simulink model "TosAeb"  (R2021a)
settings: Solver=FixedStepDiscrete  StartTime=0.0  StopTime=inf  FixedStep=0.01  RelTol=1e-3
undefined symbols (not defined in the file or in its folder): ACCModSts, AccSts1, AEB_ObjectMovingOrientation, AEB_ObjectMovingState, CN_BTCActive, CN_CurrentGearR, CN_CurrentGearR_DHT, CN_PTCActive, CN_VDCActive, CN_VSC_EspOff, CN_VSC_FCW_AEB_Error, CN_VSC_FCW_AEB_NoDispay, CN_VSC_FCW_AEB_PerfmDgdtn, CN_VSC_FCW_AEB_TempUnAvlbl, CN_VSC_VehStandstill, CNaccelAvoidMax, CNaccelAvoidMin, CNbrakePedalPress, CNcomfortStrLeftAccelMax, CNcomfortStrLeftAccelMin, CNcomfortStrRightAccelMax, CNcomfortStrRightAccelMin, CNDrvDoorOpen, CNDrvModeEco, CNDrvModeNorm, CNDrvModeOther, CNDrvModeSport, CNDrvSBRreminder, CNNull, CNobjInvalidID, CNPassDoorOpen, CNPassSBRreminder, CNPEBSAccelNegInf, CNPEBSAccelNull, CNPEBSTimeInf, CNPEBSTimeNull, CNSTOPMAX, CNSTOPNULL, CNsubjWidth, CNSwtOFF, CNSwtON, CNTTCMAX, CNTTCNULL, CNVehConfigAEBCycNone, CNVehConfigAEBNone, CNVehConfigAEBPedNone, CNVehConfigFCWNone, CNVehConfigJANone, CollisionSeverity, FALSE, FCWSnvty, JACycObjCalcInfoDefaultParams, JAPedObjCalcInfoDefaultParams, JAVehObjCalcInfoDefaultParams, latCycObjCalcInfoDefaultParams, latPedObjCalcInfoDefaultParams, longObjCalcInfoDefaultParams, ModSts, movingStatus, ObjectType ... (+1112)
libraries: simulink x3031, AEBSFunctionLib x50, Ta_Library x33, AEBSStateMachineLib x23, Mylibrary x12, simulink_extras x7, ObjSelect_lib x3, dspstat3 x2
(part 83 of 102)

== system TosAeb/FcwAebJa_Step_sys/ALG_AEBSystem/ALG_AEBController/AEB_FuncImpManagementApp/FuncManeuArbCalc/brkFuncManeuArbCalc/axTgtReqValueCalc1/axTgtReqValueCalc4AebControl/aDesiredCalc/If Action Subsystem1/accelAvoidLowLvlBrkCalc/Situation1/Case2 ==
  note: vEgo^2
      | aAvoid = ----------------------------------
      |                   vObj^2
      |                  ---------  - 2(dRel - dScure)
      |                   aObj
  subjVel : Inport
  vxAbsObj : Inport  Port=2
  axAbsObj : Inport  Port=3
  dxEgoBrake : Inport  Port=4
  Action Port : ActionPort  ActionPortLabel=else
  Divide : Product  Inputs=*/
  Divide1 : Product  Inputs=*/
  Gain : Gain  Gain=2
  Math Function : Math  Operator=square  SignedPower=on  SaturateOnIntegerOverflow=on
  Math Function1 : Math  Operator=square  SignedPower=on  SaturateOnIntegerOverflow=on
  Subtract : Sum  Inputs=+-
  accelAvoid : Outport
  wires:
  Divide1/1 -> accelAvoid/1
  Math Function1/1 -> Divide1/1
  Subtract/1 -> Divide1/2
  subjVel/1 -> Math Function1/1
  Gain/1 -> Subtract/2
  dxEgoBrake/1 -> Gain/1
  Divide/1 -> Subtract/1
  axAbsObj/1 -> Divide/2
  Math Function/1 -> Divide/1
  vxAbsObj/1 -> Math Function/1

== system TosAeb/FcwAebJa_Step_sys/ALG_AEBSystem/ALG_AEBController/AEB_FuncImpManagementApp/FuncManeuArbCalc/brkFuncManeuArbCalc/axTgtReqValueCalc1/axTgtReqValueCalc4AebControl/aDesiredCalc/If Action Subsystem1/accelAvoidLowLvlBrkCalc/Situation1/collisionFirstFlgCalc ==
  note: Calculate Ego and Obj Collision Time and
      |  Determine if the Collision Time is less than the object stop time
  dxEgoBrake : Inport
  vx : Inport  Port=2
  tObjStop : Inport  Port=3
  CNTTCMAX : Constant  Value=CNTTCMAX
  Divide : Product  Inputs=*/
  Gain : Gain  Gain=-1
  Gain1 : Gain  Gain=2
  MinMax : MinMax  Inputs=2
  Relational Operator : RelationalOperator  Operator=<  OutDataTypeStr=boolean  RndMeth=Simplest
  collisionFirstFlg : Outport
  wires:
  CNTTCMAX/1 -> MinMax/2
  MinMax/1 -> Relational Operator/1
  Relational Operator/1 -> collisionFirstFlg/1  "collisionFirstFlg"
  tObjStop/1 -> Relational Operator/2
  Gain1/1 -> MinMax/1
  Gain/1 -> Divide/2
  vx/1 -> Gain/1
  dxEgoBrake/1 -> Divide/1
  Divide/1 -> Gain1/1

== system TosAeb/FcwAebJa_Step_sys/ALG_AEBSystem/ALG_AEBController/AEB_JaObjCalcApp/JAPedTgtInfoCalc/JAPedObjCalcInfoCalc/JAPedObjInfoCalc/ObjCrossFlgCal/CollisionProbCalc/Subsystem/PreCrossFlgCalcFlow/VectorCrossAlgorithm/If Action Subsystem1/Subsystem ==
  temp_u : Inport  OutDataTypeStr=single
  temp_t : Inport  Port=2  OutDataTypeStr=single
  vectorMulit : Inport  Port=3
  Compare To Constant : simulink/Logic and Bit Operations/Compare To Constant  relop=>=  const=0  OutDataTypeStr=boolean
  Compare To Constant1 : simulink/Logic and Bit Operations/Compare To Constant  relop=<=  const=1  OutDataTypeStr=boolean
  Compare To Constant2 : simulink/Logic and Bit Operations/Compare To Constant  relop=>=  const=0  OutDataTypeStr=boolean
  Compare To Constant3 : simulink/Logic and Bit Operations/Compare To Constant  relop=<=  const=1  OutDataTypeStr=boolean
  Divide : Product  Inputs=*/
  Divide1 : Product  Inputs=*/
  Logical Operator : Logic  Inputs=4  AllPortsSameDT=off  OutDataTypeStr=boolean
  CrossFlg : Outport
  wires:
  vectorMulit/1 -> Divide1/2, Divide/2
  temp_t/1 -> Divide/1
  temp_u/1 -> Divide1/1
  Divide1/1 -> Compare To Constant1/1, Compare To Constant/1
  Divide/1 -> Compare To Constant3/1, Compare To Constant2/1
  Compare To Constant1/1 -> Logical Operator/2
  Compare To Constant2/1 -> Logical Operator/3
  Compare To Constant/1 -> Logical Operator/1
  Compare To Constant3/1 -> Logical Operator/4
  Logical Operator/1 -> CrossFlg/1

== system TosAeb/FcwAebJa_Step_sys/ALG_AEBSystem/ALG_AEBController/AEB_JaObjCalcApp/JAPedTgtInfoCalc/JAPedObjCalcInfoCalc/JAPedObjInfoCalc/ObjCrossFlgCal/CollisionProbCalc/Subsystem/PreCrossFlgCalcFlow/VectorCrossAlgorithm/If Action Subsystem1/t ==
  m2 : Inport  OutDataTypeStr=single
  m1 : Inport  Port=2  OutDataTypeStr=single
  y1 : Inport  Port=3  OutDataTypeStr=single
  n1 : Inport  Port=4  OutDataTypeStr=single
  n2 : Inport  Port=5  OutDataTypeStr=single
  x1 : Inport  Port=6  OutDataTypeStr=single
  Product : Product  OutDataTypeStr=single
  Product1 : Product  OutDataTypeStr=single
  Subtract{1..5} (5 alike) : Sum  Inputs=+-  OutDataTypeStr=single
  temp_t : Outport
  wires:
  m2/1 -> Subtract2/1
  m1/1 -> Subtract2/2, Subtract1/2
  y1/1 -> Subtract3/1
  Subtract2/1 -> Product/1
  Subtract3/1 -> Product/2
  n2/1 -> Subtract4/1
  n1/1 -> Subtract4/2, Subtract3/2
  x1/1 -> Subtract1/1
  Subtract4/1 -> Product1/1
  Subtract1/1 -> Product1/2
  Product/1 -> Subtract5/1
  Product1/1 -> Subtract5/2
  Subtract5/1 -> temp_t/1

== system TosAeb/FcwAebJa_Step_sys/ALG_AEBSystem/ALG_AEBController/AEB_JaObjCalcApp/JAPedTgtInfoCalc/JAPedObjCalcInfoCalc/JAPedObjInfoCalc/ObjCrossFlgCal/CollisionProbCalc/Subsystem/PreCrossFlgCalcFlow/VectorCrossAlgorithm/If Action Subsystem1/u ==
  x2 : Inport  OutDataTypeStr=single
  x1 : Inport  Port=2  OutDataTypeStr=single
  y1 : Inport  Port=3  OutDataTypeStr=single
  n1 : Inport  Port=4  OutDataTypeStr=single
  y2 : Inport  Port=5  OutDataTypeStr=single
  m1 : Inport  Port=6  OutDataTypeStr=single
  Product : Product  OutDataTypeStr=single
  Product1 : Product  OutDataTypeStr=single
  Subtract{1..5} (5 alike) : Sum  Inputs=+-  OutDataTypeStr=single
  temp_u : Outport
  wires:
  Product1/1 -> Subtract5/2
  Product/1 -> Subtract5/1
  Subtract1/1 -> Product1/2
  Subtract4/1 -> Product1/1
  n1/1 -> Subtract3/2
  y2/1 -> Subtract4/1
  Subtract3/1 -> Product/2
  Subtract2/1 -> Product/1
  y1/1 -> Subtract4/2, Subtract3/1
  x1/1 -> Subtract1/1, Subtract2/2
  x2/1 -> Subtract2/1
  m1/1 -> Subtract1/2
  Subtract5/1 -> temp_u/1

== system TosAeb/FcwAebJa_Step_sys/ALG_AEBSystem/ALG_AEBController/AEB_JaObjCalcApp/JAVehTgtInfoCalc/AEBJAVehObjCalc/JAVehObjInfoCalc/ObjCrossFlgCal/CollisionProbCalc/Subsystem/PreCrossFlgCalcFlow/VectorCrossAlgorithm/If Action Subsystem1/Subsystem ==
  temp_u : Inport  OutDataTypeStr=single
  temp_t : Inport  Port=2  OutDataTypeStr=single
  vectorMulit : Inport  Port=3
  Compare To Constant : simulink/Logic and Bit Operations/Compare To Constant  relop=>=  const=0  OutDataTypeStr=boolean
  Compare To Constant1 : simulink/Logic and Bit Operations/Compare To Constant  relop=<=  const=1  OutDataTypeStr=boolean
  Compare To Constant2 : simulink/Logic and Bit Operations/Compare To Constant  relop=>=  const=0  OutDataTypeStr=boolean
  Compare To Constant3 : simulink/Logic and Bit Operations/Compare To Constant  relop=<=  const=1  OutDataTypeStr=boolean
  Divide : Product  Inputs=*/
  Divide1 : Product  Inputs=*/
  Logical Operator : Logic  Inputs=4  AllPortsSameDT=off  OutDataTypeStr=boolean
  CrossFlg : Outport
  wires:
  vectorMulit/1 -> Divide1/2, Divide/2
  temp_t/1 -> Divide/1
  temp_u/1 -> Divide1/1
  Divide1/1 -> Compare To Constant1/1, Compare To Constant/1
  Divide/1 -> Compare To Constant3/1, Compare To Constant2/1
  Compare To Constant1/1 -> Logical Operator/2
  Compare To Constant2/1 -> Logical Operator/3
  Compare To Constant/1 -> Logical Operator/1
  Compare To Constant3/1 -> Logical Operator/4
  Logical Operator/1 -> CrossFlg/1

== system TosAeb/FcwAebJa_Step_sys/ALG_AEBSystem/ALG_AEBController/AEB_JaObjCalcApp/JAVehTgtInfoCalc/AEBJAVehObjCalc/JAVehObjInfoCalc/ObjCrossFlgCal/CollisionProbCalc/Subsystem/PreCrossFlgCalcFlow/VectorCrossAlgorithm/If Action Subsystem1/t ==
  m2 : Inport  OutDataTypeStr=single
  m1 : Inport  Port=2  OutDataTypeStr=single
  y1 : Inport  Port=3  OutDataTypeStr=single
  n1 : Inport  Port=4  OutDataTypeStr=single
  n2 : Inport  Port=5  OutDataTypeStr=single
  x1 : Inport  Port=6  OutDataTypeStr=single
  Product : Product  OutDataTypeStr=single
  Product1 : Product  OutDataTypeStr=single
  Subtract{1..5} (5 alike) : Sum  Inputs=+-  OutDataTypeStr=single
  temp_t : Outport
  wires:
  m2/1 -> Subtract2/1
  m1/1 -> Subtract2/2, Subtract1/2
  y1/1 -> Subtract3/1
  Subtract2/1 -> Product/1
  Subtract3/1 -> Product/2
  n2/1 -> Subtract4/1
  n1/1 -> Subtract4/2, Subtract3/2
  x1/1 -> Subtract1/1
  Subtract4/1 -> Product1/1
  Subtract1/1 -> Product1/2
  Product/1 -> Subtract5/1
  Product1/1 -> Subtract5/2
  Subtract5/1 -> temp_t/1

== system TosAeb/FcwAebJa_Step_sys/ALG_AEBSystem/ALG_AEBController/AEB_JaObjCalcApp/JAVehTgtInfoCalc/AEBJAVehObjCalc/JAVehObjInfoCalc/ObjCrossFlgCal/CollisionProbCalc/Subsystem/PreCrossFlgCalcFlow/VectorCrossAlgorithm/If Action Subsystem1/u ==
  x2 : Inport  OutDataTypeStr=single
  x1 : Inport  Port=2  OutDataTypeStr=single
  y1 : Inport  Port=3  OutDataTypeStr=single
  n1 : Inport  Port=4  OutDataTypeStr=single
  y2 : Inport  Port=5  OutDataTypeStr=single
  m1 : Inport  Port=6  OutDataTypeStr=single
  Product : Product  OutDataTypeStr=single
  Product1 : Product  OutDataTypeStr=single
  Subtract{1..5} (5 alike) : Sum  Inputs=+-  OutDataTypeStr=single
  temp_u : Outport
  wires:
  Product1/1 -> Subtract5/2
  Product/1 -> Subtract5/1
  Subtract1/1 -> Product1/2
  Subtract4/1 -> Product1/1
  n1/1 -> Subtract3/2
  y2/1 -> Subtract4/1
  Subtract3/1 -> Product/2
  Subtract2/1 -> Product/1
  y1/1 -> Subtract4/2, Subtract3/1
  x1/1 -> Subtract1/1, Subtract2/2
  x2/1 -> Subtract2/1
  m1/1 -> Subtract1/2
  Subtract5/1 -> temp_u/1

== system TosAeb/FcwAebJa_Step_sys/ALG_AEBSystem/ALG_AEBController/AEB_JaObjCalcApp/JaCycTgtInfoCalc/JaCycObjCalcInfoCalc/JaCycObjInfoCalc/ObjCrossFlgCal/CollisionProbCalc/Subsystem/PreCrossFlgCalcFlow/VectorCrossAlgorithm/If Action Subsystem1/Subsystem ==
  temp_u : Inport  OutDataTypeStr=single
  temp_t : Inport  Port=2  OutDataTypeStr=single
  vectorMulit : Inport  Port=3
  Compare To Constant : simulink/Logic and Bit Operations/Compare To Constant  relop=>=  const=0  OutDataTypeStr=boolean
  Compare To Constant1 : simulink/Logic and Bit Operations/Compare To Constant  relop=<=  const=1  OutDataTypeStr=boolean
  Compare To Constant2 : simulink/Logic and Bit Operations/Compare To Constant  relop=>=  const=0  OutDataTypeStr=boolean
  Compare To Constant3 : simulink/Logic and Bit Operations/Compare To Constant  relop=<=  const=1  OutDataTypeStr=boolean
  Divide : Product  Inputs=*/
  Divide1 : Product  Inputs=*/
  Logical Operator : Logic  Inputs=4  AllPortsSameDT=off  OutDataTypeStr=boolean
  CrossFlg : Outport
  wires:
  vectorMulit/1 -> Divide1/2, Divide/2
  temp_t/1 -> Divide/1
  temp_u/1 -> Divide1/1
  Divide1/1 -> Compare To Constant1/1, Compare To Constant/1
  Divide/1 -> Compare To Constant3/1, Compare To Constant2/1
  Compare To Constant1/1 -> Logical Operator/2
  Compare To Constant2/1 -> Logical Operator/3
  Compare To Constant/1 -> Logical Operator/1
  Compare To Constant3/1 -> Logical Operator/4
  Logical Operator/1 -> CrossFlg/1

== system TosAeb/FcwAebJa_Step_sys/ALG_AEBSystem/ALG_AEBController/AEB_JaObjCalcApp/JaCycTgtInfoCalc/JaCycObjCalcInfoCalc/JaCycObjInfoCalc/ObjCrossFlgCal/CollisionProbCalc/Subsystem/PreCrossFlgCalcFlow/VectorCrossAlgorithm/If Action Subsystem1/t ==
  m2 : Inport  OutDataTypeStr=single
  m1 : Inport  Port=2  OutDataTypeStr=single
  y1 : Inport  Port=3  OutDataTypeStr=single
  n1 : Inport  Port=4  OutDataTypeStr=single
  n2 : Inport  Port=5  OutDataTypeStr=single
  x1 : Inport  Port=6  OutDataTypeStr=single
  Product : Product  OutDataTypeStr=single
  Product1 : Product  OutDataTypeStr=single
  Subtract{1..5} (5 alike) : Sum  Inputs=+-  OutDataTypeStr=single
  temp_t : Outport
  wires:
  m2/1 -> Subtract2/1
  m1/1 -> Subtract2/2, Subtract1/2
  y1/1 -> Subtract3/1
  Subtract2/1 -> Product/1
  Subtract3/1 -> Product/2
  n2/1 -> Subtract4/1
  n1/1 -> Subtract4/2, Subtract3/2
  x1/1 -> Subtract1/1
  Subtract4/1 -> Product1/1
  Subtract1/1 -> Product1/2
  Product/1 -> Subtract5/1
  Product1/1 -> Subtract5/2
  Subtract5/1 -> temp_t/1

== system TosAeb/FcwAebJa_Step_sys/ALG_AEBSystem/ALG_AEBController/AEB_JaObjCalcApp/JaCycTgtInfoCalc/JaCycObjCalcInfoCalc/JaCycObjInfoCalc/ObjCrossFlgCal/CollisionProbCalc/Subsystem/PreCrossFlgCalcFlow/VectorCrossAlgorithm/If Action Subsystem1/u ==
  x2 : Inport  OutDataTypeStr=single
  x1 : Inport  Port=2  OutDataTypeStr=single
  y1 : Inport  Port=3  OutDataTypeStr=single
  n1 : Inport  Port=4  OutDataTypeStr=single
  y2 : Inport  Port=5  OutDataTypeStr=single
  m1 : Inport  Port=6  OutDataTypeStr=single
  Product : Product  OutDataTypeStr=single
  Product1 : Product  OutDataTypeStr=single
  Subtract{1..5} (5 alike) : Sum  Inputs=+-  OutDataTypeStr=single
  temp_u : Outport
  wires:
  Product1/1 -> Subtract5/2
  Product/1 -> Subtract5/1
  Subtract1/1 -> Product1/2
  Subtract4/1 -> Product1/1
  n1/1 -> Subtract3/2
  y2/1 -> Subtract4/1
  Subtract3/1 -> Product/2
  Subtract2/1 -> Product/1
  y1/1 -> Subtract4/2, Subtract3/1
  x1/1 -> Subtract1/1, Subtract2/2
  x2/1 -> Subtract2/1
  m1/1 -> Subtract1/2
  Subtract5/1 -> temp_u/1

== system TosAeb/FcwAebJa_Step_sys/ALG_AEBSystem/ALG_AEBController/AEB_LatObjInfoCalcApp/LatCycObjCalc/LatCycObjCalc/latCycObjInfoCalc/ObjColiisionRiskProbCalc/CollisionRiskProbCalc/ObjReginCorrCalc/AccelXYMaxMinCorrCalc/AccelXMaxMinCorrCalc/ObjDwn2UpMoving ==
  AccelMaxX : Inport
  AccelMinX : Inport  Port=2
  Action Port : ActionPort  ActionPortLabel=elseif(u1 > 0)
  AccelXMaxCorr : Outport
  AccelXMinCorr : Outport  Port=2
  wires:
  AccelMaxX/1 -> AccelXMaxCorr/1
  AccelMinX/1 -> AccelXMinCorr/1

== system TosAeb/FcwAebJa_Step_sys/ALG_AEBSystem/ALG_AEBController/AEB_LatObjInfoCalcApp/LatCycObjCalc/LatCycObjCalc/latCycObjInfoCalc/ObjColiisionRiskProbCalc/CollisionRiskProbCalc/ObjReginCorrCalc/AccelXYMaxMinCorrCalc/AccelXMaxMinCorrCalc/ObjStanding ==
  Action Port : ActionPort  ActionPortLabel=else
  Constant : Constant  Value=CNNull
  Constant1 : Constant  Value=CNNull
  AccelXMaxCorr : Outport
  AccelXMinCorr : Outport  Port=2
  wires:
  Constant1/1 -> AccelXMinCorr/1
  Constant/1 -> AccelXMaxCorr/1

== system TosAeb/FcwAebJa_Step_sys/ALG_AEBSystem/ALG_AEBController/AEB_LatObjInfoCalcApp/LatCycObjCalc/LatCycObjCalc/latCycObjInfoCalc/ObjColiisionRiskProbCalc/CollisionRiskProbCalc/ObjReginCorrCalc/AccelXYMaxMinCorrCalc/AccelXMaxMinCorrCalc/ObjUp2DwnMoving ==
  AccelMaxX : Inport
  AccelMinX : Inport  Port=2
  Action Port : ActionPort  ActionPortLabel=if(u1 < 0)
  AccelXMaxCorr : Outport
  AccelXMinCorr : Outport  Port=2
  wires:
  AccelMaxX/1 -> AccelXMaxCorr/1
  AccelMinX/1 -> AccelXMinCorr/1

== system TosAeb/FcwAebJa_Step_sys/ALG_AEBSystem/ALG_AEBController/AEB_LatObjInfoCalcApp/LatCycObjCalc/LatCycObjCalc/latCycObjInfoCalc/ObjColiisionRiskProbCalc/CollisionRiskProbCalc/ObjReginCorrCalc/AccelXYMaxMinCorrCalc/AccelYMaxMinCorrCalc/ObjLeft2RightMoving ==
  AccelYMax : Inport
  AccelYMin : Inport  Port=2
  Action Port : ActionPort  ActionPortLabel=if(u1 < 0)
  AccelYMaxCorr : Outport
  AccelYMinCorr : Outport  Port=2
  wires:
  AccelYMax/1 -> AccelYMinCorr/1
  AccelYMin/1 -> AccelYMaxCorr/1

== system TosAeb/FcwAebJa_Step_sys/ALG_AEBSystem/ALG_AEBController/AEB_LatObjInfoCalcApp/LatCycObjCalc/LatCycObjCalc/latCycObjInfoCalc/ObjColiisionRiskProbCalc/CollisionRiskProbCalc/ObjReginCorrCalc/AccelXYMaxMinCorrCalc/AccelYMaxMinCorrCalc/ObjRight2LeftMoving ==
  AccelYMax : Inport
  AccelYMin : Inport  Port=2
  Action Port : ActionPort  ActionPortLabel=elseif(u1 > 0)
  AccelYMaxCorr : Outport
  AccelYMinCorr : Outport  Port=2
  wires:
  AccelYMin/1 -> AccelYMinCorr/1
  AccelYMax/1 -> AccelYMaxCorr/1

== system TosAeb/FcwAebJa_Step_sys/ALG_AEBSystem/ALG_AEBController/AEB_LatObjInfoCalcApp/LatCycObjCalc/LatCycObjCalc/latCycObjInfoCalc/ObjColiisionRiskProbCalc/CollisionRiskProbCalc/ObjReginCorrCalc/AccelXYMaxMinCorrCalc/AccelYMaxMinCorrCalc/ObjStanding ==
  Action Port : ActionPort  ActionPortLabel=else
  Constant : Constant  Value=CNNull
  Constant1 : Constant  Value=CNNull
  AccelYMaxCorr : Outport
  AccelYMinCorr : Outport  Port=2
  wires:
  Constant/1 -> AccelYMaxCorr/1
  Constant1/1 -> AccelYMinCorr/1

== system TosAeb/FcwAebJa_Step_sys/ALG_AEBSystem/ALG_AEBController/AEB_LatObjInfoCalcApp/LatPedObjCalc/LatPedObjCalc/latPedObjInfoCalc/ObjColiisionRiskProbCalc/CollisionRiskProbCalc/ObjReginCorrCalc/AccelXYMaxMinCorrCalc/AccelXMaxMinCorrCalc/ObjDwn2UpMoving ==
  AccelMaxX : Inport  OutDataTypeStr=single
  AccelMinX : Inport  Port=2  OutDataTypeStr=single
  Action Port : ActionPort  ActionPortLabel=elseif(u1 > 0)
  AccelXMaxCorr : Outport
  AccelXMinCorr : Outport  Port=2
  wires:
  AccelMinX/1 -> AccelXMinCorr/1
  AccelMaxX/1 -> AccelXMaxCorr/1

== system TosAeb/FcwAebJa_Step_sys/ALG_AEBSystem/ALG_AEBController/AEB_LatObjInfoCalcApp/LatPedObjCalc/LatPedObjCalc/latPedObjInfoCalc/ObjColiisionRiskProbCalc/CollisionRiskProbCalc/ObjReginCorrCalc/AccelXYMaxMinCorrCalc/AccelXMaxMinCorrCalc/ObjStanding ==
  Action Port : ActionPort  ActionPortLabel=else
  Constant : Constant  Value=0  OutDataTypeStr=single
  Constant1 : Constant  Value=0  OutDataTypeStr=single
  AccelXMaxCorr : Outport
  AccelXMinCorr : Outport  Port=2
  wires:
  Constant/1 -> AccelXMaxCorr/1
  Constant1/1 -> AccelXMinCorr/1

== system TosAeb/FcwAebJa_Step_sys/ALG_AEBSystem/ALG_AEBController/AEB_LatObjInfoCalcApp/LatPedObjCalc/LatPedObjCalc/latPedObjInfoCalc/ObjColiisionRiskProbCalc/CollisionRiskProbCalc/ObjReginCorrCalc/AccelXYMaxMinCorrCalc/AccelXMaxMinCorrCalc/ObjUp2DwnMoving ==
  AccelMaxX : Inport  OutDataTypeStr=single
  AccelMinX : Inport  Port=2  OutDataTypeStr=single
  Action Port : ActionPort  ActionPortLabel=if(u1 < 0)
  AccelXMaxCorr : Outport
  AccelXMinCorr : Outport  Port=2
  wires:
  AccelMaxX/1 -> AccelXMinCorr/1
  AccelMinX/1 -> AccelXMaxCorr/1

== system TosAeb/FcwAebJa_Step_sys/ALG_AEBSystem/ALG_AEBController/AEB_LatObjInfoCalcApp/LatPedObjCalc/LatPedObjCalc/latPedObjInfoCalc/ObjColiisionRiskProbCalc/CollisionRiskProbCalc/ObjReginCorrCalc/AccelXYMaxMinCorrCalc/AccelYMaxMinCorrCalc/ObjLeft2RightMoving ==
  AccelYMax : Inport  OutDataTypeStr=single
  AccelYMin : Inport  Port=2  OutDataTypeStr=single
  Action Port : ActionPort  ActionPortLabel=if(u1 < 0)
  AccelYMaxCorr : Outport
  AccelYMinCorr : Outport  Port=2
  wires:
  AccelYMin/1 -> AccelYMaxCorr/1
  AccelYMax/1 -> AccelYMinCorr/1

== system TosAeb/FcwAebJa_Step_sys/ALG_AEBSystem/ALG_AEBController/AEB_LatObjInfoCalcApp/LatPedObjCalc/LatPedObjCalc/latPedObjInfoCalc/ObjColiisionRiskProbCalc/CollisionRiskProbCalc/ObjReginCorrCalc/AccelXYMaxMinCorrCalc/AccelYMaxMinCorrCalc/ObjRight2LeftMoving ==
  AccelYMax : Inport  OutDataTypeStr=single
  AccelYMin : Inport  Port=2  OutDataTypeStr=single
  Action Port : ActionPort  ActionPortLabel=elseif(u1 > 0)
  AccelYMaxCorr : Outport
  AccelYMinCorr : Outport  Port=2
  wires:
  AccelYMax/1 -> AccelYMaxCorr/1
  AccelYMin/1 -> AccelYMinCorr/1

== system TosAeb/FcwAebJa_Step_sys/ALG_AEBSystem/ALG_AEBController/AEB_LatObjInfoCalcApp/LatPedObjCalc/LatPedObjCalc/latPedObjInfoCalc/ObjColiisionRiskProbCalc/CollisionRiskProbCalc/ObjReginCorrCalc/AccelXYMaxMinCorrCalc/AccelYMaxMinCorrCalc/ObjStanding ==
  Action Port : ActionPort  ActionPortLabel=else
  Constant : Constant  Value=0  OutDataTypeStr=single
  Constant1 : Constant  Value=0  OutDataTypeStr=single
  AccelYMaxCorr : Outport
  AccelYMinCorr : Outport  Port=2
  wires:
  Constant1/1 -> AccelYMinCorr/1
  Constant/1 -> AccelYMaxCorr/1

== system TosAeb/FcwAebJa_Step_sys/ALG_AEBSystem/ALG_AEBController/AEB_LatObjInfoCalcApp/LatPedObjCalc/Subsystem/latPedObjInfoCalc/ObjColiisionRiskProbCalc/CollisionRiskProbCalc/ObjReginCorrCalc/AccelXYMaxMinCorrCalc/AccelXMaxMinCorrCalc/ObjDwn2UpMoving ==
  AccelMaxX : Inport  OutDataTypeStr=single
  AccelMinX : Inport  Port=2  OutDataTypeStr=single
  Action Port : ActionPort  ActionPortLabel=elseif(u1 > 0)
  AccelXMaxCorr : Outport
  AccelXMinCorr : Outport  Port=2
  wires:
  AccelMinX/1 -> AccelXMinCorr/1
  AccelMaxX/1 -> AccelXMaxCorr/1

== system TosAeb/FcwAebJa_Step_sys/ALG_AEBSystem/ALG_AEBController/AEB_LatObjInfoCalcApp/LatPedObjCalc/Subsystem/latPedObjInfoCalc/ObjColiisionRiskProbCalc/CollisionRiskProbCalc/ObjReginCorrCalc/AccelXYMaxMinCorrCalc/AccelXMaxMinCorrCalc/ObjStanding ==
  Action Port : ActionPort  ActionPortLabel=else
  Constant : Constant  Value=0  OutDataTypeStr=single
  Constant1 : Constant  Value=0  OutDataTypeStr=single
  AccelXMaxCorr : Outport
  AccelXMinCorr : Outport  Port=2
  wires:
  Constant/1 -> AccelXMaxCorr/1
  Constant1/1 -> AccelXMinCorr/1

== system TosAeb/FcwAebJa_Step_sys/ALG_AEBSystem/ALG_AEBController/AEB_LatObjInfoCalcApp/LatPedObjCalc/Subsystem/latPedObjInfoCalc/ObjColiisionRiskProbCalc/CollisionRiskProbCalc/ObjReginCorrCalc/AccelXYMaxMinCorrCalc/AccelXMaxMinCorrCalc/ObjUp2DwnMoving ==
  AccelMaxX : Inport  OutDataTypeStr=single
  AccelMinX : Inport  Port=2  OutDataTypeStr=single
  Action Port : ActionPort  ActionPortLabel=if(u1 < 0)
  AccelXMaxCorr : Outport
  AccelXMinCorr : Outport  Port=2
  wires:
  AccelMaxX/1 -> AccelXMinCorr/1
  AccelMinX/1 -> AccelXMaxCorr/1

== system TosAeb/FcwAebJa_Step_sys/ALG_AEBSystem/ALG_AEBController/AEB_LatObjInfoCalcApp/LatPedObjCalc/Subsystem/latPedObjInfoCalc/ObjColiisionRiskProbCalc/CollisionRiskProbCalc/ObjReginCorrCalc/AccelXYMaxMinCorrCalc/AccelYMaxMinCorrCalc/ObjLeft2RightMoving ==
  AccelYMax : Inport  OutDataTypeStr=single
  AccelYMin : Inport  Port=2  OutDataTypeStr=single
  Action Port : ActionPort  ActionPortLabel=if(u1 < 0)
  AccelYMaxCorr : Outport
  AccelYMinCorr : Outport  Port=2
  wires:
  AccelYMin/1 -> AccelYMaxCorr/1
  AccelYMax/1 -> AccelYMinCorr/1

== system TosAeb/FcwAebJa_Step_sys/ALG_AEBSystem/ALG_AEBController/AEB_LatObjInfoCalcApp/LatPedObjCalc/Subsystem/latPedObjInfoCalc/ObjColiisionRiskProbCalc/CollisionRiskProbCalc/ObjReginCorrCalc/AccelXYMaxMinCorrCalc/AccelYMaxMinCorrCalc/ObjRight2LeftMoving ==
  AccelYMax : Inport  OutDataTypeStr=single
  AccelYMin : Inport  Port=2  OutDataTypeStr=single
  Action Port : ActionPort  ActionPortLabel=elseif(u1 > 0)
  AccelYMaxCorr : Outport
  AccelYMinCorr : Outport  Port=2
  wires:
  AccelYMax/1 -> AccelYMaxCorr/1
  AccelYMin/1 -> AccelYMinCorr/1

== system TosAeb/FcwAebJa_Step_sys/ALG_AEBSystem/ALG_AEBController/AEB_LatObjInfoCalcApp/LatPedObjCalc/Subsystem/latPedObjInfoCalc/ObjColiisionRiskProbCalc/CollisionRiskProbCalc/ObjReginCorrCalc/AccelXYMaxMinCorrCalc/AccelYMaxMinCorrCalc/ObjStanding ==
  Action Port : ActionPort  ActionPortLabel=else
  Constant : Constant  Value=0  OutDataTypeStr=single
  Constant1 : Constant  Value=0  OutDataTypeStr=single
  AccelYMaxCorr : Outport
  AccelYMinCorr : Outport  Port=2
  wires:
  Constant1/1 -> AccelYMinCorr/1
  Constant/1 -> AccelYMaxCorr/1

== system TosAeb/FcwAebJa_Step_sys/ALG_AEBSystem/ALG_AEBController/AEB_LongTgtInfoCalc/LongObjCalcInfoCalc/AEBS_LongCollisionAssesApp/LongCollisionAvoidAssess/HighLvlBrkCalc/HighLvlBrkCalc4Brake/accelAvoidHighLvlBrkSituation1/accelAvoidLowLvlBrkCalc/Situation1/Case1 ==
  axAbsObj : Inport
  vx : Inport  Port=2
  dxEgoBrake : Inport  Port=3
  Action Port : ActionPort  ActionPortLabel=if(u1)
  Divide : Product  Inputs=*/
  Gain : Gain  Gain=2
  Math Function : Math  Operator=square  SignedPower=on  SaturateOnIntegerOverflow=on
  Subtract : Sum  Inputs=+-
  accelAvoid : Outport
  wires:
  vx/1 -> Math Function/1
  Math Function/1 -> Divide/1
  Gain/1 -> Divide/2
  dxEgoBrake/1 -> Gain/1
  Divide/1 -> Subtract/2
  axAbsObj/1 -> Subtract/1
  Subtract/1 -> accelAvoid/1

== system TosAeb/FcwAebJa_Step_sys/ALG_AEBSystem/ALG_AEBController/AEB_LongTgtInfoCalc/LongObjCalcInfoCalc/AEBS_LongCollisionAssesApp/LongCollisionAvoidAssess/HighLvlBrkCalc/HighLvlBrkCalc4Brake/accelAvoidHighLvlBrkSituation1/accelAvoidLowLvlBrkCalc/Situation1/Case2 ==
  note: vEgo^2
      | aAvoid = ----------------------------------
      |                   vObj^2
      |                  ---------  - 2(dRel - dScure)
      |                   aObj
  subjVel : Inport
  vxAbsObj : Inport  Port=2
  axAbsObj : Inport  Port=3
  dxEgoBrake : Inport  Port=4
  Action Port : ActionPort  ActionPortLabel=else
  Divide : Product  Inputs=*/
  Divide1 : Product  Inputs=*/
  Gain : Gain  Gain=2
  Math Function : Math  Operator=square  SignedPower=on  SaturateOnIntegerOverflow=on
  Math Function1 : Math  Operator=square  SignedPower=on  SaturateOnIntegerOverflow=on
  Subtract : Sum  Inputs=+-
  accelAvoid : Outport
  wires:
  vxAbsObj/1 -> Math Function/1
  Math Function/1 -> Divide/1
  axAbsObj/1 -> Divide/2
  Divide/1 -> Subtract/1
  dxEgoBrake/1 -> Gain/1
  Gain/1 -> Subtract/2
  subjVel/1 -> Math Function1/1
  Subtract/1 -> Divide1/2
  Math Function1/1 -> Divide1/1
  Divide1/1 -> accelAvoid/1

== system TosAeb/FcwAebJa_Step_sys/ALG_AEBSystem/ALG_AEBController/AEB_LongTgtInfoCalc/LongObjCalcInfoCalc/AEBS_LongCollisionAssesApp/LongCollisionAvoidAssess/HighLvlBrkCalc/HighLvlBrkCalc4Brake/accelAvoidHighLvlBrkSituation1/accelAvoidLowLvlBrkCalc/Situation1/collisionFirstFlgCalc ==
  note: Calculate Ego and Obj Collision Time and
      |  Determine if the Collision Time is less than the object stop time
  dxEgoBrake : Inport
  vx : Inport  Port=2
  tObjStop : Inport  Port=3
  CNTTCMAX : Constant  Value=CNTTCMAX
  Divide : Product  Inputs=*/
  Gain : Gain  Gain=-1
  Gain1 : Gain  Gain=2
  MinMax : MinMax  Inputs=2
  Relational Operator : RelationalOperator  Operator=<  OutDataTypeStr=boolean  RndMeth=Simplest
  collisionFirstFlg : Outport
  wires:
  Divide/1 -> Gain1/1
  dxEgoBrake/1 -> Divide/1
  vx/1 -> Gain/1
  Gain/1 -> Divide/2
  Gain1/1 -> MinMax/1
  tObjStop/1 -> Relational Operator/2
  Relational Operator/1 -> collisionFirstFlg/1  "collisionFirstFlg"
  MinMax/1 -> Relational Operator/1
  CNTTCMAX/1 -> MinMax/2

== system TosAeb/FcwAebJa_Step_sys/ALG_AEBSystem/ALG_AEBController/AEB_LongTgtInfoCalc/LongObjCalcInfoCalc/AEBS_LongCollisionAssesApp/LongCollisionAvoidAssess/HighLvlBrkCalc/HighLvlBrkCalc4Brake/accelAvoidHighLvlBrkSituation1/accelAvoidLowLvlBrkCalc2/Situation1/Case1 ==
  axAbsObj : Inport
  vx : Inport  Port=2
  dxEgoBrake : Inport  Port=3
  Action Port : ActionPort
  Divide : Product  Inputs=*/
  Gain : Gain  Gain=2
  Math Function : Math  Operator=square  SignedPower=on  SaturateOnIntegerOverflow=on
  Subtract : Sum  Inputs=+-
  accelAvoid : Outport
  wires:
  Subtract/1 -> accelAvoid/1
  axAbsObj/1 -> Subtract/1
  Divide/1 -> Subtract/2
  dxEgoBrake/1 -> Gain/1
  Gain/1 -> Divide/2
  Math Function/1 -> Divide/1
  vx/1 -> Math Function/1

== system TosAeb/FcwAebJa_Step_sys/ALG_AEBSystem/ALG_AEBController/AEB_LongTgtInfoCalc/LongObjCalcInfoCalc/AEBS_LongCollisionAssesApp/LongCollisionAvoidAssess/HighLvlBrkCalc/HighLvlBrkCalc4Brake/accelAvoidHighLvlBrkSituation1/accelAvoidLowLvlBrkCalc2/Situation1/Case2 ==
  note: vEgo^2
      | aAvoid = ----------------------------------
      |                   vObj^2
      |                  ---------  - 2(dRel - dScure)
      |                   aObj
  subjVel : Inport
  vxAbsObj : Inport  Port=2
  axAbsObj : Inport  Port=3
  dxEgoBrake : Inport  Port=4
  Action Port : ActionPort
  Divide : Product  Inputs=*/
  Divide1 : Product  Inputs=*/
  Gain : Gain  Gain=2
  Math Function : Math  Operator=square  SignedPower=on  SaturateOnIntegerOverflow=on
  Math Function1 : Math  Operator=square  SignedPower=on  SaturateOnIntegerOverflow=on
  Subtract : Sum  Inputs=+-
  accelAvoid : Outport
  wires:
  Divide1/1 -> accelAvoid/1
  Math Function1/1 -> Divide1/1
  Subtract/1 -> Divide1/2
  subjVel/1 -> Math Function1/1
  Gain/1 -> Subtract/2
  dxEgoBrake/1 -> Gain/1
  Divide/1 -> Subtract/1
  axAbsObj/1 -> Divide/2
  Math Function/1 -> Divide/1
  vxAbsObj/1 -> Math Function/1

== system TosAeb/FcwAebJa_Step_sys/ALG_AEBSystem/ALG_AEBController/AEB_LongTgtInfoCalc/LongObjCalcInfoCalc/AEBS_LongCollisionAssesApp/LongCollisionAvoidAssess/HighLvlBrkCalc/HighLvlBrkCalc4Brake/accelAvoidHighLvlBrkSituation1/accelAvoidLowLvlBrkCalc2/Situation1/collisionFirstFlgCalc ==
  note: Calculate Ego and Obj Collision Time and
      |  Determine if the Collision Time is less than the object stop time
  dxEgoBrake : Inport
  vx : Inport  Port=2
  tObjStop : Inport  Port=3
  Divide : Product  Inputs=*/
  Gain : Gain  Gain=-1
  Gain1 : Gain  Gain=2
  Relational Operator : RelationalOperator  Operator=<  OutDataTypeStr=boolean  RndMeth=Simplest
  Saturation : Saturate  UpperLimit=CNTTCMAX  LowerLimit=CNTTCNULL
      settings: AttributesFormatString=|
      | UpperLimit:%<UpperLimit>
      | LowerLimit:%<LowerLimit>
  collisionFirstFlg : Outport
  wires:
  Saturation/1 -> Relational Operator/1
  Relational Operator/1 -> collisionFirstFlg/1
  tObjStop/1 -> Relational Operator/2
  Gain1/1 -> Saturation/1
  Gain/1 -> Divide/2
  vx/1 -> Gain/1
  dxEgoBrake/1 -> Divide/1
  Divide/1 -> Gain1/1

== system TosAeb/FcwAebJa_Step_sys/ALG_AEBSystem/ALG_AEBController/AEB_LongTgtInfoCalc/LongObjCalcInfoCalc/AEBS_LongCollisionAssesApp/LongCollisionAvoidAssess/HighLvlBrkCalc/HighLvlBrkCalc4Brake/accelAvoidHighLvlBrkSituation2/accelAvoidHighLvlBrkCalc/If Action Subsystem1/accelAvoidLowLvlBrkCalc ==
  vx : Inport
  axAbsObj : Inport  Port=2
  vxAbsObj : Inport  Port=3
  subjVel : Inport  Port=4
  tObjStop : Inport  Port=5
  dxEgoBrake : Inport  Port=6
  AccelAvoidSituationJudge : Stateflow chart  TreatAsAtomicUnit=on
  Bus Creator : BusCreator  Inputs=6
  Bus Selector : BusSelector  OutputSignals=vx,axAbsObj
  Bus Selector1 : BusSelector  OutputSignals=dxEgoBrake,vx,tObjStop,subjVel,vxAbsObj,axAbsObj
  Bus Selector2 : BusSelector  OutputSignals=axAbsObj,vx,dxEgoBrake
  Bus Selector3 : BusSelector  OutputSignals=subjVel,vxAbsObj,axAbsObj,dxEgoBrake
  Merge : Merge  Inputs=4
  Situation1 : Subsystem  TreatAsAtomicUnit=on
  Situation2 : Subsystem  TreatAsAtomicUnit=on
  Situation3 : Subsystem  TreatAsAtomicUnit=on
  Situation4 : Subsystem  TreatAsAtomicUnit=on
  accelAvoidLowLvlBrkLimit : Subsystem
  accelAvoidLowLvlBrk : Outport
  wires:
  Bus Selector1/6 -> Situation1/6(axAbsObj)  "<axAbsObj>"
  Bus Selector1/5 -> Situation1/5(vxAbsObj)  "<vxAbsObj>"
  Bus Selector1/4 -> Situation1/4(subjVel)  "<subjVel>"
  Bus Selector1/3 -> Situation1/3(tObjStop)  "<tObjStop>"
  Bus Selector1/2 -> Situation1/2(vx)  "<vx>"
  Bus Selector1/1 -> Situation1/1(dxEgoBrake)  "<dxEgoBrake>"
  AccelAvoidSituationJudge/1(Situation1()) -> Situation1/Trigger
  Situation1/1(accelAvoidLowLvlBrk) -> Merge/1
  Situation2/1(accelAvoidLowLvlBrk) -> Merge/2
  Situation3/1(accelAvoidLowLvlBrk) -> Merge/3
  Situation4/1(accelAvoidLowLvlBrk) -> Merge/4
  AccelAvoidSituationJudge/2(Situation2()) -> [Situation2]
  AccelAvoidSituationJudge/3(Situation3()) -> [Situation3]
  AccelAvoidSituationJudge/4(Situation4()) -> [Situation4]
  [Situation2] -> Situation2/Trigger
  [Situation3] -> Situation3/Trigger
  [Situation4] -> Situation4/Trigger
  vxAbsObj/1 -> Bus Creator/3  "vxAbsObj"
  subjVel/1 -> Bus Creator/4  "subjVel"
  dxEgoBrake/1 -> Bus Creator/6  "dxEgoBrake"
  vx/1 -> Bus Creator/1  "vx"
  axAbsObj/1 -> Bus Creator/2  "axAbsObj"
  tObjStop/1 -> Bus Creator/5  "tObjStop"
  Bus Creator/1 -> Bus Selector3/1, Bus Selector2/1, Bus Selector1/1, Bus Selector/1
  Bus Selector/1 -> AccelAvoidSituationJudge/1(vx)  "<vx>"
  Bus Selector/2 -> AccelAvoidSituationJudge/2(axAbsObj)  "<axAbsObj>"
  Bus Selector2/3 -> Situation2/3(dxEgoBrake)  "<dxEgoBrake>"
  Bus Selector2/2 -> Situation2/2(vx)  "<vx>"
  Bus Selector2/1 -> Situation2/1(axAbsObj)  "<axAbsObj>"
  Bus Selector3/1 -> Situation3/1(subjVel)  "<subjVel>"
  Bus Selector3/2 -> Situation3/2(vxAbsObj)  "<vxAbsObj>"
  Bus Selector3/3 -> Situation3/3(axAbsObj)  "<axAbsObj>"
  Bus Selector3/4 -> Situation3/4(dxEgoBrake)  "<dxEgoBrake>"
  Merge/1 -> accelAvoidLowLvlBrkLimit/1(accelAvoidLowLvlBrkNoLimit)
  accelAvoidLowLvlBrkLimit/1(accelAvoidLowLvlBrk) -> accelAvoidLowLvlBrk/1

== system TosAeb/FcwAebJa_Step_sys/ALG_AEBSystem/ALG_AEBController/AEB_LongTgtInfoCalc/LongObjCalcInfoCalc/AEBS_LongCollisionAssesApp/LongCollisionAvoidAssess/HighLvlBrkCalc/HighLvlBrkCalc4Brake/accelAvoidHighLvlBrkSituation2/accelAvoidHighLvlBrkCalc/If Action Subsystem1/accelAvoidLowLvlBrkCalc1 ==
  vx : Inport
  axAbsObj : Inport  Port=2
  vxAbsObj : Inport  Port=3
  subjVel : Inport  Port=4
  tObjStop : Inport  Port=5
  dxEgoBrake : Inport  Port=6
  AccelAvoidSituationJudge : Stateflow chart  TreatAsAtomicUnit=on
  Bus Creator : BusCreator  Inputs=6
  Bus Selector : BusSelector  OutputSignals=vxRelTargetObjCalc,axAbsTargetObjCalc
  Bus Selector1 : BusSelector  OutputSignals=dxEgoBrake,vxRelTargetObjCalc,tObjStop,subjVel,vxAbsTargetObjCalc,axAbsTargetObjCalc
  Bus Selector2 : BusSelector  OutputSignals=axAbsTargetObjCalc,vxRelTargetObjCalc,dxEgoBrake
  Bus Selector3 : BusSelector  OutputSignals=subjVel,vxAbsTargetObjCalc,axAbsTargetObjCalc,dxEgoBrake
  Merge : Merge  Inputs=4
  Situation1 : Subsystem  TreatAsAtomicUnit=on
  Situation2 : Subsystem  TreatAsAtomicUnit=on
  Situation3 : Subsystem  TreatAsAtomicUnit=on
  Situation4 : Subsystem  TreatAsAtomicUnit=on
  accelAvoidLowLvlBrkLimit : Subsystem
  accelAvoidLowLvlBrk : Outport
  wires:
  accelAvoidLowLvlBrkLimit/1(accelAvoidLowLvlBrk) -> accelAvoidLowLvlBrk/1
  Merge/1 -> accelAvoidLowLvlBrkLimit/1(accelAvoidLowLvlBrkNoLimit)
  Bus Selector3/4 -> Situation3/4(dxEgoBrake)  "<dxEgoBrake>"
  Bus Selector3/3 -> Situation3/3(axAbsObj)  "<axAbsTargetObjCalc>"
  Bus Selector3/2 -> Situation3/2(vxAbsObj)  "<vxAbsTargetObjCalc>"
  Bus Selector3/1 -> Situation3/1(subjVel)  "<subjVel>"
  Bus Selector2/1 -> Situation2/1(axAbsObj)  "<axAbsTargetObjCalc>"
  Bus Selector2/2 -> Situation2/2(vx)  "<vxRelTargetObjCalc>"
  Bus Selector2/3 -> Situation2/3(dxEgoBrake)  "<dxEgoBrake>"
  Bus Selector/2 -> AccelAvoidSituationJudge/2(axAbsTargetObjCalc)  "<axAbsTargetObjCalc>"
  Bus Selector/1 -> AccelAvoidSituationJudge/1(vxRelTargetObjCalc)  "<vxRelTargetObjCalc>"
  Bus Creator/1 -> Bus Selector/1, Bus Selector1/1, Bus Selector2/1, Bus Selector3/1
  tObjStop/1 -> Bus Creator/5  "tObjStop"
  axAbsObj/1 -> Bus Creator/2  "axAbsTargetObjCalc"
  vx/1 -> Bus Creator/1  "vxRelTargetObjCalc"
  dxEgoBrake/1 -> Bus Creator/6  "dxEgoBrake"
  subjVel/1 -> Bus Creator/4  "subjVel"
  vxAbsObj/1 -> Bus Creator/3  "vxAbsTargetObjCalc"
  [Situation4] -> Situation4/Trigger
  [Situation3] -> Situation3/Trigger
  [Situation2] -> Situation2/Trigger
  AccelAvoidSituationJudge/4(Situation4()) -> [Situation4]
  AccelAvoidSituationJudge/3(Situation3()) -> [Situation3]
  AccelAvoidSituationJudge/2(Situation2()) -> [Situation2]
  Situation4/1(accelAvoidLowLvlBrk) -> Merge/4
  Situation3/1(accelAvoidLowLvlBrk) -> Merge/3
  Situation2/1(accelAvoidLowLvlBrk) -> Merge/2
  Situation1/1(accelAvoidLowLvlBrk) -> Merge/1
  AccelAvoidSituationJudge/1(Situation1()) -> Situation1/Trigger
  Bus Selector1/1 -> Situation1/1(dxEgoBrake)  "<dxEgoBrake>"
  Bus Selector1/2 -> Situation1/2(vx)  "<vxRelTargetObjCalc>"
  Bus Selector1/3 -> Situation1/3(tObjStop)  "<tObjStop>"
  Bus Selector1/4 -> Situation1/4(subjVel)  "<subjVel>"
  Bus Selector1/5 -> Situation1/5(vxAbsObj)  "<vxAbsTargetObjCalc>"
  Bus Selector1/6 -> Situation1/6(axAbsObj)  "<axAbsTargetObjCalc>"

== system TosAeb/FcwAebJa_Step_sys/ALG_AEBSystem/ALG_AEBController/AEB_LongTgtInfoCalc/LongObjCalcInfoCalc/AEBS_LongCollisionAssesApp/LongCollisionAvoidAssess/HighLvlBrkCalc/HighLvlBrkCalc4Brake/accelAvoidHighLvlBrkSituation2/accelAvoidHighLvlBrkCalc/tObjStopCalc/StopTimeCalc ==
  velRel : Inport
  accelRel : Inport  Port=2
  tStopFuncCalc : TriggerPort  TriggerType=function-call  VariantControl=Variant
  Abs : Abs
  Abs1 : Abs
  Divide : Product  Inputs=*/
  tStop : Outport
  wires:
  Divide/1 -> tStop/1
  Abs1/1 -> Divide/2
  Abs/1 -> Divide/1
  accelRel/1 -> Abs1/1
  velRel/1 -> Abs/1

== system TosAeb/FcwAebJa_Step_sys/ALG_AEBSystem/ALG_AEBController/AEB_LongTgtInfoCalc/LongObjCalcInfoCalc/AEBS_LongCollisionAssesApp/LongCollisionAvoidAssess/HighLvlBrkCalc/HighLvlBrkCalc4Brake/accelAvoidHighLvlBrkSituation3/If Action Subsystem1/DistReactionCalc/If Action Subsystem ==
  Action Port : ActionPort  ActionPortLabel=if(u1)
  Constant : Constant  Value=CNNull
  dxObjReaction : Outport
  wires:
  Constant/1 -> dxObjReaction/1

== system TosAeb/FcwAebJa_Step_sys/ALG_AEBSystem/ALG_AEBController/AEB_LongTgtInfoCalc/LongObjCalcInfoCalc/AEBS_LongCollisionAssesApp/LongCollisionAvoidAssess/HighLvlBrkCalc/HighLvlBrkCalc4Brake/accelAvoidHighLvlBrkSituation3/If Action Subsystem1/DistReactionCalc/If Action Subsystem1 ==
  vxAbsObj : Inport
  axAbsObj : Inport  Port=2
  Action Port : ActionPort  ActionPortLabel=else
  Divide : Product  Inputs=*/
  Gain : Gain  Gain=-2
  Math Function : Math  Operator=square  SignedPower=on  SaturateOnIntegerOverflow=on
  dxObjReaction : Outport
  wires:
  vxAbsObj/1 -> Math Function/1
  Math Function/1 -> Divide/1
  axAbsObj/1 -> Gain/1
  Gain/1 -> Divide/2
  Divide/1 -> dxObjReaction/1

== system TosAeb/FcwAebJa_Step_sys/ALG_AEBSystem/ALG_AEBController/AEB_LongTgtInfoCalc/LongObjCalcInfoCalc/AEBS_LongCollisionAssesApp/LongCollisionAvoidAssess/HighLvlBrkCalc/HighLvlBrkCalc4Brake/accelAvoidHighLvlBrkSituation3/If Action Subsystem1/accelAvoidHighLvlBrkCalc/MovingSateCalcAfterReactionTime ==
  note: For Situation 3,after driver reaction time ,the object has been stopped.
      | Ego vehicle is keeping moving.
  subjLongAccel : Inport
  tReaction : Inport  Port=2
  subjVel : Inport  Port=3
  Add2 : Sum
  Constant : Constant  Value=CNNull
  Product3 : Product
  Subtract : Sum  Inputs=+-
  vxRelTargetPredict : Outport
  axAbsTargetPredict : Outport  Port=2
  vxAbsTargetPredict : Outport  Port=3
  subjVelPredict : Outport  Port=4
  tObjStopPredict : Outport  Port=5
  wires:
  subjVel/1 -> Add2/2
  Product3/1 -> Add2/1
  subjLongAccel/1 -> Product3/1
  tReaction/1 -> Product3/2
  Constant/1 -> Subtract/1, vxAbsTargetPredict/1, tObjStopPredict/1, axAbsTargetPredict/1
  Subtract/1 -> vxRelTargetPredict/1
  Add2/1 -> Subtract/2, subjVelPredict/1

== system TosAeb/FcwAebJa_Step_sys/ALG_AEBSystem/ALG_AEBController/AEB_LongTgtInfoCalc/LongObjCalcInfoCalc/AEBS_LongCollisionAssesApp/LongCollisionAvoidAssess/HighLvlBrkCalc/HighLvlBrkCalc4Brake/accelAvoidHighLvlBrkSituation3/If Action Subsystem1/accelAvoidHighLvlBrkCalc/accelAvoidLowLvlBrkCalc ==
  vx : Inport
  axAbsObj : Inport  Port=2
  vxAbsObj : Inport  Port=3
  subjVel : Inport  Port=4
  tObjStop : Inport  Port=5
  dxEgoBrake : Inport  Port=6
  AccelAvoidSituationJudge : Stateflow chart  TreatAsAtomicUnit=on
  Bus Creator : BusCreator  Inputs=6
  Bus Selector : BusSelector  OutputSignals=vx,axAbsObj
  Bus Selector1 : BusSelector  OutputSignals=dxEgoBrake,vx,tObjStop,subjVel,vxAbsObj,axAbsObj
  Bus Selector2 : BusSelector  OutputSignals=axAbsObj,vx,dxEgoBrake
  Bus Selector3 : BusSelector  OutputSignals=subjVel,vxAbsObj,axAbsObj,dxEgoBrake
  Merge : Merge  Inputs=4
  Situation1 : Subsystem  TreatAsAtomicUnit=on
  Situation2 : Subsystem  TreatAsAtomicUnit=on
  Situation3 : Subsystem  TreatAsAtomicUnit=on
  Situation4 : Subsystem  TreatAsAtomicUnit=on
  accelAvoidLowLvlBrkLimit : Subsystem
  accelAvoidLowLvlBrk : Outport
  wires:
  Bus Selector1/6 -> Situation1/6(axAbsObj)  "<axAbsObj>"
  Bus Selector1/5 -> Situation1/5(vxAbsObj)  "<vxAbsObj>"
  Bus Selector1/4 -> Situation1/4(subjVel)  "<subjVel>"
  Bus Selector1/3 -> Situation1/3(tObjStop)  "<tObjStop>"
  Bus Selector1/2 -> Situation1/2(vx)  "<vx>"
  Bus Selector1/1 -> Situation1/1(dxEgoBrake)  "<dxEgoBrake>"
  AccelAvoidSituationJudge/1(Situation1()) -> Situation1/Trigger
  Situation1/1(accelAvoidLowLvlBrk) -> Merge/1
  Situation2/1(accelAvoidLowLvlBrk) -> Merge/2
  Situation3/1(accelAvoidLowLvlBrk) -> Merge/3
  Situation4/1(accelAvoidLowLvlBrk) -> Merge/4
  AccelAvoidSituationJudge/2(Situation2()) -> [Situation2]
  AccelAvoidSituationJudge/3(Situation3()) -> [Situation3]
  AccelAvoidSituationJudge/4(Situation4()) -> [Situation4]
  [Situation2] -> Situation2/Trigger
  [Situation3] -> Situation3/Trigger
  [Situation4] -> Situation4/Trigger
  vxAbsObj/1 -> Bus Creator/3  "vxAbsObj"
  subjVel/1 -> Bus Creator/4  "subjVel"
  dxEgoBrake/1 -> Bus Creator/6  "dxEgoBrake"
  vx/1 -> Bus Creator/1  "vx"
  axAbsObj/1 -> Bus Creator/2  "axAbsObj"
  tObjStop/1 -> Bus Creator/5  "tObjStop"
  Bus Creator/1 -> Bus Selector3/1, Bus Selector2/1, Bus Selector1/1, Bus Selector/1
  Bus Selector/1 -> AccelAvoidSituationJudge/1(vx)  "<vx>"
  Bus Selector/2 -> AccelAvoidSituationJudge/2(axAbsObj)  "<axAbsObj>"
  Bus Selector2/3 -> Situation2/3(dxEgoBrake)  "<dxEgoBrake>"
  Bus Selector2/2 -> Situation2/2(vx)  "<vx>"
  Bus Selector2/1 -> Situation2/1(axAbsObj)  "<axAbsObj>"
  Bus Selector3/1 -> Situation3/1(subjVel)  "<subjVel>"
  Bus Selector3/2 -> Situation3/2(vxAbsObj)  "<vxAbsObj>"
  Bus Selector3/3 -> Situation3/3(axAbsObj)  "<axAbsObj>"
  Bus Selector3/4 -> Situation3/4(dxEgoBrake)  "<dxEgoBrake>"
  Merge/1 -> accelAvoidLowLvlBrkLimit/1(accelAvoidLowLvlBrkNoLimit)
  accelAvoidLowLvlBrkLimit/1(accelAvoidLowLvlBrk) -> accelAvoidLowLvlBrk/1

== system TosAeb/FcwAebJa_Step_sys/ALG_AEBSystem/ALG_AEBController/AEB_LongTgtInfoCalc/LongObjCalcInfoCalc/AEBS_LongCollisionAssesApp/LongCollisionAvoidAssess/HighLvlBrkCalc/HighLvlBrkCalc4Brake/accelAvoidHighLvlBrkSituation3/If Action Subsystem1/accelAvoidHighLvlBrkCalc/accelAvoidLowLvlBrkCalc1 ==
  vx : Inport
  axAbsObj : Inport  Port=2
  vxAbsObj : Inport  Port=3
  subjVel : Inport  Port=4
  tObjStop : Inport  Port=5
  dxEgoBrake : Inport  Port=6
  AccelAvoidSituationJudge : Stateflow chart  TreatAsAtomicUnit=on
  Bus Creator : BusCreator  Inputs=6
  Bus Selector : BusSelector  OutputSignals=vxRelTargetObjCalc,axAbsTargetObjCalc
  Bus Selector1 : BusSelector  OutputSignals=dxEgoBrake,vxRelTargetObjCalc,tObjStop,subjVel,vxAbsTargetObjCalc,axAbsTargetObjCalc
  Bus Selector2 : BusSelector  OutputSignals=axAbsTargetObjCalc,vxRelTargetObjCalc,dxEgoBrake
  Bus Selector3 : BusSelector  OutputSignals=subjVel,vxAbsTargetObjCalc,axAbsTargetObjCalc,dxEgoBrake
  Merge : Merge  Inputs=4
  Situation1 : Subsystem  TreatAsAtomicUnit=on
  Situation2 : Subsystem  TreatAsAtomicUnit=on
  Situation3 : Subsystem  TreatAsAtomicUnit=on
  Situation4 : Subsystem  TreatAsAtomicUnit=on
  accelAvoidLowLvlBrkLimit : Subsystem
  accelAvoidLowLvlBrk : Outport
  wires:
  accelAvoidLowLvlBrkLimit/1(accelAvoidLowLvlBrk) -> accelAvoidLowLvlBrk/1
  Merge/1 -> accelAvoidLowLvlBrkLimit/1(accelAvoidLowLvlBrkNoLimit)
  Bus Selector3/4 -> Situation3/4(dxEgoBrake)  "<dxEgoBrake>"
  Bus Selector3/3 -> Situation3/3(axAbsObj)  "<axAbsTargetObjCalc>"
  Bus Selector3/2 -> Situation3/2(vxAbsObj)  "<vxAbsTargetObjCalc>"
  Bus Selector3/1 -> Situation3/1(subjVel)  "<subjVel>"
  Bus Selector2/1 -> Situation2/1(axAbsObj)  "<axAbsTargetObjCalc>"
  Bus Selector2/2 -> Situation2/2(vx)  "<vxRelTargetObjCalc>"
  Bus Selector2/3 -> Situation2/3(dxEgoBrake)  "<dxEgoBrake>"
  Bus Selector/2 -> AccelAvoidSituationJudge/2(axAbsTargetObjCalc)  "<axAbsTargetObjCalc>"
  Bus Selector/1 -> AccelAvoidSituationJudge/1(vxRelTargetObjCalc)  "<vxRelTargetObjCalc>"
  Bus Creator/1 -> Bus Selector/1, Bus Selector1/1, Bus Selector2/1, Bus Selector3/1
  tObjStop/1 -> Bus Creator/5  "tObjStop"
  axAbsObj/1 -> Bus Creator/2  "axAbsTargetObjCalc"
  vx/1 -> Bus Creator/1  "vxRelTargetObjCalc"
  dxEgoBrake/1 -> Bus Creator/6  "dxEgoBrake"
  subjVel/1 -> Bus Creator/4  "subjVel"
  vxAbsObj/1 -> Bus Creator/3  "vxAbsTargetObjCalc"
  [Situation4] -> Situation4/Trigger
  [Situation3] -> Situation3/Trigger
  [Situation2] -> Situation2/Trigger
  AccelAvoidSituationJudge/4(Situation4()) -> [Situation4]
  AccelAvoidSituationJudge/3(Situation3()) -> [Situation3]
  AccelAvoidSituationJudge/2(Situation2()) -> [Situation2]
  Situation4/1(accelAvoidLowLvlBrk) -> Merge/4
  Situation3/1(accelAvoidLowLvlBrk) -> Merge/3
  Situation2/1(accelAvoidLowLvlBrk) -> Merge/2
  Situation1/1(accelAvoidLowLvlBrk) -> Merge/1
  AccelAvoidSituationJudge/1(Situation1()) -> Situation1/Trigger
  Bus Selector1/1 -> Situation1/1(dxEgoBrake)  "<dxEgoBrake>"
  Bus Selector1/2 -> Situation1/2(vx)  "<vxRelTargetObjCalc>"
  Bus Selector1/3 -> Situation1/3(tObjStop)  "<tObjStop>"
  Bus Selector1/4 -> Situation1/4(subjVel)  "<subjVel>"
  Bus Selector1/5 -> Situation1/5(vxAbsObj)  "<vxAbsTargetObjCalc>"
  Bus Selector1/6 -> Situation1/6(axAbsObj)  "<axAbsTargetObjCalc>"

== system TosAeb/FcwAebJa_Step_sys/ALG_AEBSystem/ALG_AEBController/AEB_LongTgtInfoCalc/LongObjCalcInfoCalc/AEBS_LongCollisionAssesApp/LongCollisionAvoidAssess/HighLvlBrkCalc/HighLvlBrkCalc4Brake/accelAvoidHighLvlBrkSituation5/If Action Subsystem1/If Action Subsystem1/If Action Subsystem2 ==
  Action Port : ActionPort  ActionPortLabel=if(u1)
  Constant : Constant  Value=CNNull
  dxObjReaction : Outport
  wires:
  Constant/1 -> dxObjReaction/1

== system TosAeb/FcwAebJa_Step_sys/ALG_AEBSystem/ALG_AEBController/AEB_LongTgtInfoCalc/LongObjCalcInfoCalc/AEBS_LongCollisionAssesApp/LongCollisionAvoidAssess/HighLvlBrkCalc/HighLvlBrkCalc4Brake/accelAvoidHighLvlBrkSituation5/If Action Subsystem1/If Action Subsystem1/If Action Subsystem3 ==
  vxAbsObj : Inport
  axAbsObj : Inport  Port=2
  Action Port : ActionPort  ActionPortLabel=else
  Divide : Product  Inputs=*/
  Gain : Gain  Gain=-2
  Math Function : Math  Operator=square  SignedPower=on  SaturateOnIntegerOverflow=on
  dxObjReaction : Outport
  wires:
  vxAbsObj/1 -> Math Function/1
  Math Function/1 -> Divide/1
  axAbsObj/1 -> Gain/1
  Gain/1 -> Divide/2
  Divide/1 -> dxObjReaction/1

== system TosAeb/FcwAebJa_Step_sys/ALG_AEBSystem/ALG_AEBController/AEB_LongTgtInfoCalc/LongObjCalcInfoCalc/AEBS_LongCollisionAssesApp/LongCollisionAvoidAssess/HighLvlBrkCalc/HighLvlBrkCalc4Warning/accelAvoidHighLvlBrkSituation1/accelAvoidLowLvlBrkCalc/Situation1/Case1 ==
  axAbsObj : Inport
  vx : Inport  Port=2
  dxEgoBrake : Inport  Port=3
  Action Port : ActionPort  ActionPortLabel=if(u1)
  Divide : Product  Inputs=*/
  Gain : Gain  Gain=2
  Math Function : Math  Operator=square  SignedPower=on  SaturateOnIntegerOverflow=on
  Subtract : Sum  Inputs=+-
  accelAvoid : Outport
  wires:
  Subtract/1 -> accelAvoid/1
  axAbsObj/1 -> Subtract/1
  Divide/1 -> Subtract/2
  dxEgoBrake/1 -> Gain/1
  Gain/1 -> Divide/2
  Math Function/1 -> Divide/1
  vx/1 -> Math Function/1

== system TosAeb/FcwAebJa_Step_sys/ALG_AEBSystem/ALG_AEBController/AEB_LongTgtInfoCalc/LongObjCalcInfoCalc/AEBS_LongCollisionAssesApp/LongCollisionAvoidAssess/HighLvlBrkCalc/HighLvlBrkCalc4Warning/accelAvoidHighLvlBrkSituation1/accelAvoidLowLvlBrkCalc/Situation1/Case2 ==
  note: vEgo^2
      | aAvoid = ----------------------------------
      |                   vObj^2
      |                  ---------  - 2(dRel - dScure)
      |                   aObj
  subjVel : Inport
  vxAbsObj : Inport  Port=2
  axAbsObj : Inport  Port=3
  dxEgoBrake : Inport  Port=4
  Action Port : ActionPort  ActionPortLabel=else
  Divide : Product  Inputs=*/
  Divide1 : Product  Inputs=*/
  Gain : Gain  Gain=2
  Math Function : Math  Operator=square  SignedPower=on  SaturateOnIntegerOverflow=on
  Math Function1 : Math  Operator=square  SignedPower=on  SaturateOnIntegerOverflow=on
  Subtract : Sum  Inputs=+-
  accelAvoid : Outport
  wires:
  Divide1/1 -> accelAvoid/1
  Math Function1/1 -> Divide1/1
  Subtract/1 -> Divide1/2
  subjVel/1 -> Math Function1/1
  Gain/1 -> Subtract/2
  dxEgoBrake/1 -> Gain/1
  Divide/1 -> Subtract/1
  axAbsObj/1 -> Divide/2
  Math Function/1 -> Divide/1
  vxAbsObj/1 -> Math Function/1

== system TosAeb/FcwAebJa_Step_sys/ALG_AEBSystem/ALG_AEBController/AEB_LongTgtInfoCalc/LongObjCalcInfoCalc/AEBS_LongCollisionAssesApp/LongCollisionAvoidAssess/HighLvlBrkCalc/HighLvlBrkCalc4Warning/accelAvoidHighLvlBrkSituation1/accelAvoidLowLvlBrkCalc/Situation1/collisionFirstFlgCalc ==
  note: Calculate Ego and Obj Collision Time and
      |  Determine if the Collision Time is less than the object stop time
  dxEgoBrake : Inport
  vx : Inport  Port=2
  tObjStop : Inport  Port=3
  CNTTCMAX : Constant  Value=CNTTCMAX
  Divide : Product  Inputs=*/
  Gain : Gain  Gain=-1
  Gain1 : Gain  Gain=2
  MinMax : MinMax  Inputs=2
  Relational Operator : RelationalOperator  Operator=<  OutDataTypeStr=boolean  RndMeth=Simplest
  collisionFirstFlg : Outport
  wires:
  CNTTCMAX/1 -> MinMax/2
  MinMax/1 -> Relational Operator/1
  Relational Operator/1 -> collisionFirstFlg/1  "collisionFirstFlg"
  tObjStop/1 -> Relational Operator/2
  Gain1/1 -> MinMax/1
  Gain/1 -> Divide/2
  vx/1 -> Gain/1
  dxEgoBrake/1 -> Divide/1
  Divide/1 -> Gain1/1
